simulink model "dds_cwg_32k_dual_test"  (R2016b) (continued)
== system dds_cwg_32k_dual_test/nb_pfb == (continued)
      mask Display:
      | port_label('input', 1, 'd');
      | port_label('output', 1, 'q');
      | disp(['Z^-', num2str(latency)]);
  pipeline61 : Subsystem  Commented=on  AncestorBlock=casper_library_delays/pipeline  mask(pipeline){latency=2}
      mask help:
      | An explicitly laid-out delay line to avoid mapping to SRL16s
      | for use in pipelining to help achieve timing closure.
      mask init:
      | pipeline_init(gcb,'latency',latency);
      settings: AttributesFormatString=Latency = %<latency>
      mask Display:
      | port_label('input', 1, 'd');
      | port_label('output', 1, 'q');
      | disp(['Z^-', num2str(latency)]);
  pipeline62 : Subsystem  AncestorBlock=casper_library_delays/pipeline  mask(pipeline){latency=2}
      mask help:
      | An explicitly laid-out delay line to avoid mapping to SRL16s
      | for use in pipelining to help achieve timing closure.
      mask init:
      | pipeline_init(gcb,'latency',latency);
      settings: AttributesFormatString=Latency = %<latency>
      mask Display:
      | port_label('input', 1, 'd');
      | port_label('output', 1, 'q');
      | disp(['Z^-', num2str(latency)]);
  pipeline63 : Subsystem  AncestorBlock=casper_library_delays/pipeline  mask(pipeline){latency=2}
      mask help:
      | An explicitly laid-out delay line to avoid mapping to SRL16s
      | for use in pipelining to help achieve timing closure.
      mask init:
      | pipeline_init(gcb,'latency',latency);
      settings: AttributesFormatString=Latency = %<latency>
      mask Display:
      | port_label('input', 1, 'd');
      | port_label('output', 1, 'q');
      | disp(['Z^-', num2str(latency)]);
  pipeline64 : Subsystem  AncestorBlock=casper_library_delays/pipeline  mask(pipeline){latency=2}
      mask help:
      | An explicitly laid-out delay line to avoid mapping to SRL16s
      | for use in pipelining to help achieve timing closure.
      mask init:
      | pipeline_init(gcb,'latency',latency);
      settings: AttributesFormatString=Latency = %<latency>
      mask Display:
      | port_label('input', 1, 'd');
      | port_label('output', 1, 'q');
      | disp(['Z^-', num2str(latency)]);
  pipeline65 : Subsystem  AncestorBlock=casper_library_delays/pipeline  mask(pipeline){latency=2}
      mask help:
      | An explicitly laid-out delay line to avoid mapping to SRL16s
      | for use in pipelining to help achieve timing closure.
      mask init:
      | pipeline_init(gcb,'latency',latency);
      settings: AttributesFormatString=Latency = %<latency>
      mask Display:
      | port_label('input', 1, 'd');
      | port_label('output', 1, 'q');
      | disp(['Z^-', num2str(latency)]);
  pipeline66 : Subsystem  AncestorBlock=casper_library_delays/pipeline  mask(pipeline){latency=2}
      mask help:
      | An explicitly laid-out delay line to avoid mapping to SRL16s
      | for use in pipelining to help achieve timing closure.
      mask init:
      | pipeline_init(gcb,'latency',latency);
      settings: AttributesFormatString=Latency = %<latency>
      mask Display:
      | port_label('input', 1, 'd');
      | port_label('output', 1, 'q');
      | disp(['Z^-', num2str(latency)]);
  pipeline67 : Subsystem  AncestorBlock=casper_library_delays/pipeline  mask(pipeline){latency=2}
      mask help:
      | An explicitly laid-out delay line to avoid mapping to SRL16s
      | for use in pipelining to help achieve timing closure.
      mask init:
      | pipeline_init(gcb,'latency',latency);
      settings: AttributesFormatString=Latency = %<latency>
      mask Display:
      | port_label('input', 1, 'd');
      | port_label('output', 1, 'q');
      | disp(['Z^-', num2str(latency)]);
  pipeline68 : Subsystem  AncestorBlock=casper_library_delays/pipeline  mask(pipeline){latency=2}
      mask help:
      | An explicitly laid-out delay line to avoid mapping to SRL16s
      | for use in pipelining to help achieve timing closure.
      mask init:
      | pipeline_init(gcb,'latency',latency);
      settings: AttributesFormatString=Latency = %<latency>
      mask Display:
      | port_label('input', 1, 'd');
      | port_label('output', 1, 'q');
      | disp(['Z^-', num2str(latency)]);
  pipeline69 : Subsystem  Commented=on  AncestorBlock=casper_library_delays/pipeline  mask(pipeline){latency=2}
      mask help:
      | An explicitly laid-out delay line to avoid mapping to SRL16s
      | for use in pipelining to help achieve timing closure.
      mask init:
      | pipeline_init(gcb,'latency',latency);
      settings: AttributesFormatString=Latency = %<latency>
      mask Display:
      | port_label('input', 1, 'd');
      | port_label('output', 1, 'q');
      | disp(['Z^-', num2str(latency)]);
  pipeline7 : Subsystem  AncestorBlock=casper_library_delays/pipeline  mask(pipeline){latency=2}
      mask help:
      | An explicitly laid-out delay line to avoid mapping to SRL16s
      | for use in pipelining to help achieve timing closure.
      mask init:
      | pipeline_init(gcb,'latency',latency);
      settings: AttributesFormatString=Latency = %<latency>
      mask Display:
      | port_label('input', 1, 'd');
      | port_label('output', 1, 'q');
      | disp(['Z^-', num2str(latency)]);
  pipeline70 : Subsystem  Commented=on  AncestorBlock=casper_library_delays/pipeline  mask(pipeline){latency=2}
      mask help:
      | An explicitly laid-out delay line to avoid mapping to SRL16s
      | for use in pipelining to help achieve timing closure.
      mask init:
      | pipeline_init(gcb,'latency',latency);
      settings: AttributesFormatString=Latency = %<latency>
      mask Display:
      | port_label('input', 1, 'd');
      | port_label('output', 1, 'q');
      | disp(['Z^-', num2str(latency)]);
  pipeline71 : Subsystem  AncestorBlock=casper_library_delays/pipeline  mask(pipeline){latency=2}
      mask help:
      | An explicitly laid-out delay line to avoid mapping to SRL16s
      | for use in pipelining to help achieve timing closure.
      mask init:
      | pipeline_init(gcb,'latency',latency);
      settings: AttributesFormatString=Latency = %<latency>
      mask Display:
      | port_label('input', 1, 'd');
      | port_label('output', 1, 'q');
      | disp(['Z^-', num2str(latency)]);
  pipeline72 : Subsystem  AncestorBlock=casper_library_delays/pipeline  mask(pipeline){latency=2}
      mask help:
      | An explicitly laid-out delay line to avoid mapping to SRL16s
      | for use in pipelining to help achieve timing closure.
      mask init:
      | pipeline_init(gcb,'latency',latency);
      settings: AttributesFormatString=Latency = %<latency>
      mask Display:
      | port_label('input', 1, 'd');
      | port_label('output', 1, 'q');
      | disp(['Z^-', num2str(latency)]);
  pipeline73 : Subsystem  AncestorBlock=casper_library_delays/pipeline  mask(pipeline){latency=2}
      mask help:
      | An explicitly laid-out delay line to avoid mapping to SRL16s
      | for use in pipelining to help achieve timing closure.
      mask init:
      | pipeline_init(gcb,'latency',latency);
      settings: AttributesFormatString=Latency = %<latency>
      mask Display:
      | port_label('input', 1, 'd');
      | port_label('output', 1, 'q');
      | disp(['Z^-', num2str(latency)]);
  pipeline74 : Subsystem  AncestorBlock=casper_library_delays/pipeline  mask(pipeline){latency=2}
      mask help:
      | An explicitly laid-out delay line to avoid mapping to SRL16s
      | for use in pipelining to help achieve timing closure.
      mask init:
      | pipeline_init(gcb,'latency',latency);
      settings: AttributesFormatString=Latency = %<latency>
      mask Display:
      | port_label('input', 1, 'd');
      | port_label('output', 1, 'q');
      | disp(['Z^-', num2str(latency)]);
  pipeline75 : Subsystem  AncestorBlock=casper_library_delays/pipeline  mask(pipeline){latency=2}
      mask help:
      | An explicitly laid-out delay line to avoid mapping to SRL16s
      | for use in pipelining to help achieve timing closure.
      mask init:
      | pipeline_init(gcb,'latency',latency);
      settings: AttributesFormatString=Latency = %<latency>
      mask Display:
      | port_label('input', 1, 'd');
      | port_label('output', 1, 'q');
      | disp(['Z^-', num2str(latency)]);
  pipeline76 : Subsystem  AncestorBlock=casper_library_delays/pipeline  mask(pipeline){latency=1}
      mask help:
      | An explicitly laid-out delay line to avoid mapping to SRL16s
      | for use in pipelining to help achieve timing closure.
      mask init:
      | pipeline_init(gcb,'latency',latency);
      settings: AttributesFormatString=Latency = %<latency>
      mask Display:
      | port_label('input', 1, 'd');
      | port_label('output', 1, 'q');
      | disp(['Z^-', num2str(latency)]);
  pipeline77 : Subsystem  AncestorBlock=casper_library_delays/pipeline  mask(pipeline){latency=2}
      mask help:
      | An explicitly laid-out delay line to avoid mapping to SRL16s
      | for use in pipelining to help achieve timing closure.
      mask init:
      | pipeline_init(gcb,'latency',latency);
      settings: AttributesFormatString=Latency = %<latency>
      mask Display:
      | port_label('input', 1, 'd');
      | port_label('output', 1, 'q');
      | disp(['Z^-', num2str(latency)]);
  pipeline78 : Subsystem  AncestorBlock=casper_library_delays/pipeline  mask(pipeline){latency=2}
      mask help:
      | An explicitly laid-out delay line to avoid mapping to SRL16s
      | for use in pipelining to help achieve timing closure.
      mask init:
      | pipeline_init(gcb,'latency',latency);
      settings: AttributesFormatString=Latency = %<latency>
      mask Display:
      | port_label('input', 1, 'd');
      | port_label('output', 1, 'q');
      | disp(['Z^-', num2str(latency)]);
  pipeline79 : Subsystem  Commented=on  AncestorBlock=casper_library_delays/pipeline  mask(pipeline){latency=2}
      mask help:
      | An explicitly laid-out delay line to avoid mapping to SRL16s
      | for use in pipelining to help achieve timing closure.
      mask init:
      | pipeline_init(gcb,'latency',latency);
      settings: AttributesFormatString=Latency = %<latency>
      mask Display:
      | port_label('input', 1, 'd');
      | port_label('output', 1, 'q');
      | disp(['Z^-', num2str(latency)]);
  pipeline8 : Subsystem  AncestorBlock=casper_library_delays/pipeline  mask(pipeline){latency=2}
      mask help:
      | An explicitly laid-out delay line to avoid mapping to SRL16s
      | for use in pipelining to help achieve timing closure.
      mask init:
      | pipeline_init(gcb,'latency',latency);
      settings: AttributesFormatString=Latency = %<latency>
      mask Display:
      | port_label('input', 1, 'd');
      | port_label('output', 1, 'q');
      | disp(['Z^-', num2str(latency)]);
  pipeline83 : Subsystem  AncestorBlock=casper_library_delays/pipeline  mask(pipeline){latency=2}
      mask help:
      | An explicitly laid-out delay line to avoid mapping to SRL16s
      | for use in pipelining to help achieve timing closure.
      mask init:
      | pipeline_init(gcb,'latency',latency);
      settings: AttributesFormatString=Latency = %<latency>
      mask Display:
      | port_label('input', 1, 'd');
      | port_label('output', 1, 'q');
      | disp(['Z^-', num2str(latency)]);
  pipeline9 : Subsystem  AncestorBlock=casper_library_delays/pipeline  mask(pipeline){latency=2}
      mask help:
      | An explicitly laid-out delay line to avoid mapping to SRL16s
      | for use in pipelining to help achieve timing closure.
      mask init:
      | pipeline_init(gcb,'latency',latency);
      settings: AttributesFormatString=Latency = %<latency>
      mask Display:
      | port_label('input', 1, 'd');
      | port_label('output', 1, 'q');
      | disp(['Z^-', num2str(latency)]);
  pipeline92 : Subsystem  AncestorBlock=casper_library_delays/pipeline  mask(pipeline){latency=2}
      mask help:
      | An explicitly laid-out delay line to avoid mapping to SRL16s
      | for use in pipelining to help achieve timing closure.
      mask init:
      | pipeline_init(gcb,'latency',latency);
      settings: AttributesFormatString=Latency = %<latency>
      mask Display:
      | port_label('input', 1, 'd');
      | port_label('output', 1, 'q');
      | disp(['Z^-', num2str(latency)]);
  pipeline93 : Subsystem  AncestorBlock=casper_library_delays/pipeline  mask(pipeline){latency=2}
      mask help:
      | An explicitly laid-out delay line to avoid mapping to SRL16s
      | for use in pipelining to help achieve timing closure.
      mask init:
      | pipeline_init(gcb,'latency',latency);
      settings: AttributesFormatString=Latency = %<latency>
      mask Display:
      | port_label('input', 1, 'd');
      | port_label('output', 1, 'q');
      | disp(['Z^-', num2str(latency)]);
  pipeline94 : Subsystem  AncestorBlock=casper_library_delays/pipeline  mask(pipeline){latency=2}
      mask help:
      | An explicitly laid-out delay line to avoid mapping to SRL16s
      | for use in pipelining to help achieve timing closure.
      mask init:
      | pipeline_init(gcb,'latency',latency);
      settings: AttributesFormatString=Latency = %<latency>
      mask Display:
      | port_label('input', 1, 'd');
      | port_label('output', 1, 'q');
      | disp(['Z^-', num2str(latency)]);
  pipeline95 : Subsystem  AncestorBlock=casper_library_delays/pipeline  mask(pipeline){latency=2}
      mask help:
      | An explicitly laid-out delay line to avoid mapping to SRL16s
      | for use in pipelining to help achieve timing closure.
      mask init:
      | pipeline_init(gcb,'latency',latency);
      settings: AttributesFormatString=Latency = %<latency>
      mask Display:
      | port_label('input', 1, 'd');
      | port_label('output', 1, 'q');
      | disp(['Z^-', num2str(latency)]);
  pipeline96 : Subsystem  AncestorBlock=casper_library_delays/pipeline  mask(pipeline){latency=2}
      mask help:
      | An explicitly laid-out delay line to avoid mapping to SRL16s
      | for use in pipelining to help achieve timing closure.
      mask init:
      | pipeline_init(gcb,'latency',latency);
      settings: AttributesFormatString=Latency = %<latency>
      mask Display:
      | port_label('input', 1, 'd');
      | port_label('output', 1, 'q');
      | disp(['Z^-', num2str(latency)]);
  pipeline97 : Subsystem  AncestorBlock=casper_library_delays/pipeline  mask(pipeline){latency=2}
      mask help:
      | An explicitly laid-out delay line to avoid mapping to SRL16s
      | for use in pipelining to help achieve timing closure.
      mask init:
      | pipeline_init(gcb,'latency',latency);
      settings: AttributesFormatString=Latency = %<latency>
      mask Display:
      | port_label('input', 1, 'd');
      | port_label('output', 1, 'q');
      | disp(['Z^-', num2str(latency)]);
  pipeline98 : Subsystem  AncestorBlock=casper_library_delays/pipeline  mask(pipeline){latency=2}
      mask help:
      | An explicitly laid-out delay line to avoid mapping to SRL16s
      | for use in pipelining to help achieve timing closure.
      mask init:
      | pipeline_init(gcb,'latency',latency);
      settings: AttributesFormatString=Latency = %<latency>
      mask Display:
      | port_label('input', 1, 'd');
      | port_label('output', 1, 'q');
      | disp(['Z^-', num2str(latency)]);
  pulse_ext : casper_library_misc/pulse_ext  pulse_len=10
  pulse_ext1 : casper_library_misc/pulse_ext  pulse_len=10
  register_change_detect : Subsystem  Commented=on
  ss_fft : Subsystem  Tag=casper:bitsnap  Commented=on  AncestorBlock=casper_library_scopes/bitfield_snapshot  mask(bitsnap){io_names=[re im ch dv sync of], io_widths=[18 18 15 1 1 1], io_bps=[0 0 0 0 0 0], io_types=[0 0 0 2 2 2], show_format=on, snap_storage=bram, snap_dram_dimm=2, snap_dram_clock=250, snap_nsamples=9, snap_data_width=64, snap_offset=on, snap_circap=off, snap_value=off, snap_ext_arm=off, snap_ext_circ=off, snap_provide_outputs=on, snap_use_dsp48=on, snap_delay=0, extra_names=[c d], extra_widths=[30 2], extra_bps=[0 0], extra_types=0}
      mask prompts: io_names "Names [msb...lsb]"; io_widths "Bitwidths [msb...lsb]"; io_bps "Binary pts [msb...lsb]"; io_types "Data types [msb...lsb], ufix=0, fix=1, bool=2"; show_format "Print format string?"; snap_storage "Storage medium"; snap_dram_dimm "DRAM dimm"; snap_dram_clock "DRAM clock rate"; snap_nsamples "Number of Samples (2^?)"; snap_data_width "Data width"; snap_offset "Start delay support"; snap_circap "Circular capture support"; snap_value "Extra value capture support"; snap_ext_arm "External arm support"; snap_ext_circ "External circular capture support"; snap_provide_outputs "Provide outputs"; snap_use_dsp48 "Use DSP48s to implement counters"; snap_delay "Pre-snapshot delay"; extra_names "Names [msb...lsb]"; extra_widths "Widths [msb...lsb]"; extra_bps "Binary pts [msb...lsb]"; extra_types "Data types [msb...lsb], ufix=0, fix=1, bool=2"
      mask help:
      | A wrapped snapshot block. Allows specification of inputs to a snapblock word so the toolflow can automatically set up the scope.
      | See documentation for snapshot for explanation of parameters.
      mask init:
      | bitsnap_init(gcb);
      settings: AttributesFormatString=|
      | snap(6):uf18.0,uf18.0,uf15.0,b,b,b = 54 bits\n64 wide, 512 deep
      | debugID: 42865123
      mask TypeOptions snap_storage: bram | dram
      mask Callback snap_storage: bitsnap_callback();
      mask TypeOptions snap_dram_dimm: 1 | 2 | 3 | 4
      mask TypeOptions snap_dram_clock: 200 | 250 | 266 | 300 | 333
      mask TypeOptions snap_data_width: 8 | 16 | 32 | 64 | 128
      mask Callback snap_value: bitsnap_callback();
      mask Callback snap_ext_arm: bitsnap_callback();
      mask Callback snap_ext_circ: bitsnap_callback();
      mask Callback snap_provide_outputs: bitsnap_callback();
  ss_fft3 : Subsystem  Tag=casper:bitsnap  Commented=on  AncestorBlock=casper_library_scopes/bitfield_snapshot  mask(bitsnap){io_names=[re im shift dv_in sync rst tlast dv_out ch0 re_ou sync_out dvu trdy err fifo], io_widths=[18 18 16 1 1 1 1 1 15 18 1 1 1 1 1], io_bps=[0 0 0 0 0 0 0 0 0 0 0 0 0 0 0], io_types=[0 0 0 2 2 2 2 2 0 0 2 2 2 2 2], show_format=on, snap_storage=bram, snap_dram_dimm=2, snap_dram_clock=250, snap_nsamples=10, snap_data_width=128, snap_offset=on, snap_circap=off, snap_value=off, snap_ext_arm=off, snap_ext_circ=off, snap_provide_outputs=on, snap_use_dsp48=on, snap_delay=0, extra_names=[c d], extra_widths=[30 2], extra_bps=[0 0], extra_types=0}
      mask prompts: io_names "Names [msb...lsb]"; io_widths "Bitwidths [msb...lsb]"; io_bps "Binary pts [msb...lsb]"; io_types "Data types [msb...lsb], ufix=0, fix=1, bool=2"; show_format "Print format string?"; snap_storage "Storage medium"; snap_dram_dimm "DRAM dimm"; snap_dram_clock "DRAM clock rate"; snap_nsamples "Number of Samples (2^?)"; snap_data_width "Data width"; snap_offset "Start delay support"; snap_circap "Circular capture support"; snap_value "Extra value capture support"; snap_ext_arm "External arm support"; snap_ext_circ "External circular capture support"; snap_provide_outputs "Provide outputs"; snap_use_dsp48 "Use DSP48s to implement counters"; snap_delay "Pre-snapshot delay"; extra_names "Names [msb...lsb]"; extra_widths "Widths [msb...lsb]"; extra_bps "Binary pts [msb...lsb]"; extra_types "Data types [msb...lsb], ufix=0, fix=1, bool=2"
      mask help:
      | A wrapped snapshot block. Allows specification of inputs to a snapblock word so the toolflow can automatically set up the scope.
      | See documentation for snapshot for explanation of parameters.
      mask init:
      | bitsnap_init(gcb);
      settings: AttributesFormatString=|
      | snap(15):uf18.0,uf18.0,uf16.0,b,b,b,b,b,uf15.0,uf18.0,b,b,b,b,b = 95 bits\n128 wide, 1024 deep
      | debugID: 120913192
      mask TypeOptions snap_storage: bram | dram
      mask Callback snap_storage: bitsnap_callback();
      mask TypeOptions snap_dram_dimm: 1 | 2 | 3 | 4
      mask TypeOptions snap_dram_clock: 200 | 250 | 266 | 300 | 333
      mask TypeOptions snap_data_width: 8 | 16 | 32 | 64 | 128
      mask Callback snap_value: bitsnap_callback();
      mask Callback snap_ext_arm: bitsnap_callback();
      mask Callback snap_ext_circ: bitsnap_callback();
      mask Callback snap_provide_outputs: bitsnap_callback();
  ss_fft4 : Subsystem  Tag=casper:bitsnap  Commented=on  AncestorBlock=casper_library_scopes/bitfield_snapshot  mask(bitsnap){io_names=[re_i re_o dv we rd sync empty full], io_widths=[18 18 1 1 1 1 1 1], io_bps=[0 0 0 0 0 0 0 0], io_types=[0 0 2 2 2 2 2 2], show_format=on, snap_storage=bram, snap_dram_dimm=2, snap_dram_clock=250, snap_nsamples=9, snap_data_width=64, snap_offset=on, snap_circap=off, snap_value=off, snap_ext_arm=off, snap_ext_circ=off, snap_provide_outputs=on, snap_use_dsp48=on, snap_delay=0, extra_names=[c d], extra_widths=[30 2], extra_bps=[0 0], extra_types=0}
      mask prompts: io_names "Names [msb...lsb]"; io_widths "Bitwidths [msb...lsb]"; io_bps "Binary pts [msb...lsb]"; io_types "Data types [msb...lsb], ufix=0, fix=1, bool=2"; show_format "Print format string?"; snap_storage "Storage medium"; snap_dram_dimm "DRAM dimm"; snap_dram_clock "DRAM clock rate"; snap_nsamples "Number of Samples (2^?)"; snap_data_width "Data width"; snap_offset "Start delay support"; snap_circap "Circular capture support"; snap_value "Extra value capture support"; snap_ext_arm "External arm support"; snap_ext_circ "External circular capture support"; snap_provide_outputs "Provide outputs"; snap_use_dsp48 "Use DSP48s to implement counters"; snap_delay "Pre-snapshot delay"; extra_names "Names [msb...lsb]"; extra_widths "Widths [msb...lsb]"; extra_bps "Binary pts [msb...lsb]"; extra_types "Data types [msb...lsb], ufix=0, fix=1, bool=2"
      mask help:
      | A wrapped snapshot block. Allows specification of inputs to a snapblock word so the toolflow can automatically set up the scope.
      | See documentation for snapshot for explanation of parameters.
      mask init:
      | bitsnap_init(gcb);
      settings: AttributesFormatString=|
      | snap(8):uf18.0,uf18.0,b,b,b,b,b,b = 42 bits\n64 wide, 512 deep
      | debugID: 120942983
      mask TypeOptions snap_storage: bram | dram
      mask Callback snap_storage: bitsnap_callback();
      mask TypeOptions snap_dram_dimm: 1 | 2 | 3 | 4
      mask TypeOptions snap_dram_clock: 200 | 250 | 266 | 300 | 333
      mask TypeOptions snap_data_width: 8 | 16 | 32 | 64 | 128
      mask Callback snap_value: bitsnap_callback();
      mask Callback snap_ext_arm: bitsnap_callback();
      mask Callback snap_ext_circ: bitsnap_callback();
      mask Callback snap_provide_outputs: bitsnap_callback();
  ss_fft_debug : Subsystem  Tag=casper:bitsnap  Commented=on  AncestorBlock=casper_library_scopes/bitfield_snapshot  mask(bitsnap){io_names=[fft0_re fft0_im fft1_re fft1_im trdy0 ch0 dv0 sync0 of0 trdy1 ch1 dv1 of1], io_widths=[18 18 18 18 1 15 1 1 1 1 15 1 1], io_bps=[17 17 17 17 0 0 0 0 0 0 0 0 0], io_types=[1 1 1 1 2 0 2 2 2 2 0 2 2], show_format=on, snap_storage=bram, snap_dram_dimm=2, snap_dram_clock=250, snap_nsamples=15, snap_data_width=128, snap_offset=on, snap_circap=off, snap_value=off, snap_ext_arm=off, snap_ext_circ=off, snap_provide_outputs=on, snap_use_dsp48=on, snap_delay=0, extra_names=[c d], extra_widths=[30 2], extra_bps=[0 0], extra_types=0}
      mask prompts: io_names "Names [msb...lsb]"; io_widths "Bitwidths [msb...lsb]"; io_bps "Binary pts [msb...lsb]"; io_types "Data types [msb...lsb], ufix=0, fix=1, bool=2"; show_format "Print format string?"; snap_storage "Storage medium"; snap_dram_dimm "DRAM dimm"; snap_dram_clock "DRAM clock rate"; snap_nsamples "Number of Samples (2^?)"; snap_data_width "Data width"; snap_offset "Start delay support"; snap_circap "Circular capture support"; snap_value "Extra value capture support"; snap_ext_arm "External arm support"; snap_ext_circ "External circular capture support"; snap_provide_outputs "Provide outputs"; snap_use_dsp48 "Use DSP48s to implement counters"; snap_delay "Pre-snapshot delay"; extra_names "Names [msb...lsb]"; extra_widths "Widths [msb...lsb]"; extra_bps "Binary pts [msb...lsb]"; extra_types "Data types [msb...lsb], ufix=0, fix=1, bool=2"
      mask help:
      | A wrapped snapshot block. Allows specification of inputs to a snapblock word so the toolflow can automatically set up the scope.
      | See documentation for snapshot for explanation of parameters.
      mask init:
      | bitsnap_init(gcb);
      settings: AttributesFormatString=|
      | snap(13):f18.17,f18.17,f18.17,f18.17,b,uf15.0,b,b,b,b,uf15.0,b,b = 109 bits\n128 wide, 32768 deep
      | debugID: 34277647
      mask TypeOptions snap_storage: bram | dram
      mask Callback snap_storage: bitsnap_callback();
      mask TypeOptions snap_dram_dimm: 1 | 2 | 3 | 4
      mask TypeOptions snap_dram_clock: 200 | 250 | 266 | 300 | 333
      mask TypeOptions snap_data_width: 8 | 16 | 32 | 64 | 128
      mask Callback snap_value: bitsnap_callback();
      mask Callback snap_ext_arm: bitsnap_callback();
      mask Callback snap_ext_circ: bitsnap_callback();
      mask Callback snap_provide_outputs: bitsnap_callback();
  ss_fft_debug1 : Subsystem  Tag=casper:bitsnap  Commented=on  AncestorBlock=casper_library_scopes/bitfield_snapshot  mask(bitsnap){io_names=[fft0_re fft0_im fft1_re fft1_im trdy0 ch0 dv0 sync0 of0 trdy1 ch1 dv1 of1], io_widths=[18 18 18 18 1 15 1 1 1 1 15 1 1], io_bps=[17 17 17 17 0 0 0 0 0 0 0 0 0], io_types=[1 1 1 1 2 0 2 2 2 2 0 2 2], show_format=on, snap_storage=bram, snap_dram_dimm=2, snap_dram_clock=250, snap_nsamples=15, snap_data_width=128, snap_offset=on, snap_circap=off, snap_value=off, snap_ext_arm=off, snap_ext_circ=off, snap_provide_outputs=on, snap_use_dsp48=on, snap_delay=0, extra_names=[c d], extra_widths=[30 2], extra_bps=[0 0], extra_types=0}
      mask prompts: io_names "Names [msb...lsb]"; io_widths "Bitwidths [msb...lsb]"; io_bps "Binary pts [msb...lsb]"; io_types "Data types [msb...lsb], ufix=0, fix=1, bool=2"; show_format "Print format string?"; snap_storage "Storage medium"; snap_dram_dimm "DRAM dimm"; snap_dram_clock "DRAM clock rate"; snap_nsamples "Number of Samples (2^?)"; snap_data_width "Data width"; snap_offset "Start delay support"; snap_circap "Circular capture support"; snap_value "Extra value capture support"; snap_ext_arm "External arm support"; snap_ext_circ "External circular capture support"; snap_provide_outputs "Provide outputs"; snap_use_dsp48 "Use DSP48s to implement counters"; snap_delay "Pre-snapshot delay"; extra_names "Names [msb...lsb]"; extra_widths "Widths [msb...lsb]"; extra_bps "Binary pts [msb...lsb]"; extra_types "Data types [msb...lsb], ufix=0, fix=1, bool=2"
      mask help:
      | A wrapped snapshot block. Allows specification of inputs to a snapblock word so the toolflow can automatically set up the scope.
      | See documentation for snapshot for explanation of parameters.
      mask init:
      | bitsnap_init(gcb);
      settings: AttributesFormatString=|
      | snap(13):f18.17,f18.17,f18.17,f18.17,b,uf15.0,b,b,b,b,uf15.0,b,b = 109 bits\n128 wide, 32768 deep
      | debugID: 123077310
      mask TypeOptions snap_storage: bram | dram
      mask Callback snap_storage: bitsnap_callback();
      mask TypeOptions snap_dram_dimm: 1 | 2 | 3 | 4
      mask TypeOptions snap_dram_clock: 200 | 250 | 266 | 300 | 333
      mask TypeOptions snap_data_width: 8 | 16 | 32 | 64 | 128
      mask Callback snap_value: bitsnap_callback();
      mask Callback snap_ext_arm: bitsnap_callback();
      mask Callback snap_ext_circ: bitsnap_callback();
      mask Callback snap_provide_outputs: bitsnap_callback();
  ss_fir_out : Subsystem  Tag=casper:bitsnap  Commented=on  AncestorBlock=casper_library_scopes/bitfield_snapshot  mask(bitsnap){io_names=[fir_re fir_im dv sync], io_widths=[18 18 1 1], io_bps=[0 17 0 0], io_types=[0 1 2 2], show_format=on, snap_storage=bram, snap_dram_dimm=2, snap_dram_clock=250, snap_nsamples=9, snap_data_width=64, snap_offset=on, snap_circap=off, snap_value=off, snap_ext_arm=off, snap_ext_circ=off, snap_provide_outputs=on, snap_use_dsp48=on, snap_delay=0, extra_names=[c d], extra_widths=[30 2], extra_bps=[0 0], extra_types=0}
      mask prompts: io_names "Names [msb...lsb]"; io_widths "Bitwidths [msb...lsb]"; io_bps "Binary pts [msb...lsb]"; io_types "Data types [msb...lsb], ufix=0, fix=1, bool=2"; show_format "Print format string?"; snap_storage "Storage medium"; snap_dram_dimm "DRAM dimm"; snap_dram_clock "DRAM clock rate"; snap_nsamples "Number of Samples (2^?)"; snap_data_width "Data width"; snap_offset "Start delay support"; snap_circap "Circular capture support"; snap_value "Extra value capture support"; snap_ext_arm "External arm support"; snap_ext_circ "External circular capture support"; snap_provide_outputs "Provide outputs"; snap_use_dsp48 "Use DSP48s to implement counters"; snap_delay "Pre-snapshot delay"; extra_names "Names [msb...lsb]"; extra_widths "Widths [msb...lsb]"; extra_bps "Binary pts [msb...lsb]"; extra_types "Data types [msb...lsb], ufix=0, fix=1, bool=2"
      mask help:
      | A wrapped snapshot block. Allows specification of inputs to a snapblock word so the toolflow can automatically set up the scope.
      | See documentation for snapshot for explanation of parameters.
      mask init:
      | bitsnap_init(gcb);
      settings: AttributesFormatString=|
      | snap(4):uf18.0,f18.17,b,b = 38 bits\n64 wide, 512 deep
      | debugID: 22068793
      mask TypeOptions snap_storage: bram | dram
      mask Callback snap_storage: bitsnap_callback();
      mask TypeOptions snap_dram_dimm: 1 | 2 | 3 | 4
      mask TypeOptions snap_dram_clock: 200 | 250 | 266 | 300 | 333
      mask TypeOptions snap_data_width: 8 | 16 | 32 | 64 | 128
      mask Callback snap_value: bitsnap_callback();
      mask Callback snap_ext_arm: bitsnap_callback();
      mask Callback snap_ext_circ: bitsnap_callback();
      mask Callback snap_provide_outputs: bitsnap_callback();
  sync_out : Outport
  dv_out : Outport  Port=2
  tag scope: fft_ch0=global, fft_ch1=global, fft_conf_tready_p0=global, fft_dv0=global, fft_dv1=global, fft_p0_fifo_full=global, fft_pol0_err=global, fft_pol0_of=global, fft_pol1_err=global, fft_pol1_of=global, fft_sync0=global, fft_tready_p0=global, fir_data_tlast=global, fir_dvalid_pol0=global, fir_pol0=global, fir_sync=global
  wires:
  pipeline29/1(q) -> ss_fft_debug/5(in_trdy0)
  pipeline30/1(q) -> ss_fft_debug/6(in_ch0)
  pipeline31/1(q) -> ss_fft_debug/7(in_dv0)
  pipeline33/1(q) -> ss_fft_debug/8(in_sync0)
  pipeline26/1(q) -> ss_fft_debug/9(in_of0)
  pipeline34/1(q) -> ss_fft_debug/10(in_trdy1)
  pipeline32/1(q) -> ss_fft_debug/11(in_ch1)
  pipeline15/1(q) -> ss_fft_debug/15(trig)
  pipeline35/1(q) -> ss_fft_debug/12(in_dv1)
  pipeline51/1(q) -> ss_fft_debug/13(in_of1)
  pipeline52/1(q) -> ss_fft_debug/14(we)
  From68/1 -> pipeline34/1(d)
  From72/1 -> pipeline29/1(d)
  From73/1 -> pipeline30/1(d)
  From74/1 -> pipeline52/1(d), pipeline31/1(d)
  From75/1 -> pipeline15/1(d), pipeline33/1(d)
  From76/1 -> pipeline26/1(d)
  From77/1 -> pipeline51/1(d)
  From78/1 -> pipeline32/1(d)
  From80/1 -> pipeline35/1(d)
  pipeline1/1(q) -> ss_fft_debug/4(in_fft1_im)
  pipeline2/1(q) -> ss_fft_debug/3(in_fft1_re)
  pipeline27/1(q) -> ss_fft_debug/2(in_fft0_im)
  pipeline28/1(q) -> ss_fft_debug/1(in_fft0_re)
  From2/1 -> pipeline27/1(d)
  From1/1 -> pipeline28/1(d)
  From5/1 -> pipeline2/1(d)
  From6/1 -> pipeline1/1(d)
  pipeline45/1(q) -> ss_fft_debug1/5(in_trdy0)
  pipeline49/1(q) -> ss_fft_debug1/6(in_ch0)
  pipeline54/1(q) -> ss_fft_debug1/7(in_dv0)
  pipeline56/1(q) -> ss_fft_debug1/8(in_sync0)
  pipeline41/1(q) -> ss_fft_debug1/9(in_of0)
  pipeline57/1(q) -> ss_fft_debug1/10(in_trdy1)
  pipeline55/1(q) -> ss_fft_debug1/11(in_ch1)
  pipeline38/1(q) -> ss_fft_debug1/15(trig)
  pipeline58/1(q) -> ss_fft_debug1/12(in_dv1)
  pipeline59/1(q) -> ss_fft_debug1/13(in_of1)
  pipeline60/1(q) -> ss_fft_debug1/14(we)
  From17/1 -> pipeline57/1(d)
  From18/1 -> pipeline45/1(d)
  From19/1 -> pipeline49/1(d)
  From26/1 -> pipeline54/1(d), pipeline60/1(d)
  From20/1 -> pipeline56/1(d), pipeline38/1(d)
  From22/1 -> pipeline41/1(d)
  From23/1 -> pipeline59/1(d)
  From24/1 -> pipeline55/1(d)
  From25/1 -> pipeline58/1(d)
  pipeline37/1(q) -> ss_fft_debug1/4(in_fft1_im)
  pipeline40/1(q) -> ss_fft_debug1/3(in_fft1_re)
  pipeline42/1(q) -> ss_fft_debug1/2(in_fft0_im)
  pipeline43/1(q) -> ss_fft_debug1/1(in_fft0_re)
  From11/1 -> pipeline42/1(d)
  From8/1 -> pipeline43/1(d)
  From14/1 -> pipeline40/1(d)
  From15/1 -> pipeline37/1(d)
  [fft_sync0] -> sync_out/1
  [fft_dv0] -> dv_out/1
  pfb_fir_generic0/2(pol0_out0) -> pipeline18/1(d)
  pfb_fir_generic1/2(pol0_out0) -> pipeline20/1(d)
  pfb_fir_generic0/1(sync_out) -> pipeline17/1(d)
  pfb_fir_generic1/1(sync_out) -> Terminator5/1
  pol1/1 -> pfb_fir_generic1/2(pol0_in0)
  Gateway Out6/1 -> To Workspace6/1
  Gateway Out1/1 -> To Workspace1/1
  Gateway Out9/1 -> To Workspace9/1
  Gateway Out36/1 -> To Workspace36/1
  Gateway Out39/1 -> To Workspace39/1
  pfb_fir_generic0/3(dvalid) -> pipeline19/1(d)
  pfb_fir_generic1/3(dvalid) -> pipeline21/1(d)
  sync/1 -> pfb_fir_generic0/1(sync), pfb_fir_generic1/1(sync), Gateway Out9/1
  Counter3/1 -> Relational4/1, Gateway Out53/1
  Constant9/1 -> Relational4/2
  dvalid/1 -> pfb_fir_generic0/3(en), pfb_fir_generic1/3(en), Gateway Out36/1
  Gateway Out70/1 -> To Workspace69/1
  Gateway Out71/1 -> To Workspace70/1
  [fft_re_pol0] -> Gateway Out70/1
  [fft_im_pol0] -> Gateway Out71/1
  fft_shift/1 -> [fft_shift]
  Constant2/1 -> Mux3/2
  [fft_dv0] -> Mux3/1
  Mux3/1 -> [fft_re_pol0]
  Constant1/1 -> Mux4/2
  Mux4/1 -> [fft_im_pol0]
  [fft_re_pol0_int] -> Mux3/3
  [fft_im_pol0_int] -> Mux4/3
  [fft_dv0] -> Mux4/1
  pipeline19/1(q) -> [fir_dvalid_pol0], Gateway Out1/1, Counter3/2
  pipeline20/1(q) -> Goto16/1
  pipeline21/1(q) -> Goto18/1
  pipeline18/1(q) -> [fir_pol0]
  [cnt_rst] -> pipeline230/1(d)
  Counter12/1 -> fir_sync_cnt/1(out_reg)
  pipeline229/1(q) -> Counter12/2
  pipeline230/1(q) -> Counter12/1
  "Fast Fourier Transform 9.0 "/1 -> pipeline23/1(d)
  pipeline117/1(q) -> [fft_tready_p0]
  pipeline110/1(q) -> [fft_ch0]
  Gateway Out66/1 -> To Workspace65/1
  Gateway Out67/1 -> To Workspace66/1
  Gateway Out72/1 -> To Workspace71/1
  [fft_dv0] -> Gateway Out67/1
  [fft_ch0] -> Gateway Out66/1
  [fft_tready_p0] -> Gateway Out72/1
  "Fast Fourier Transform 9.0 "/5 -> pipeline122/1(d)
  "Fast Fourier Transform 9.0 "/6 -> pipeline123/1(d)
  "Fast Fourier Transform 9.0 "/8 -> pipeline110/1(d), Relational2/1
  "Fast Fourier Transform 9.0 "/15 -> Terminator7/1
  "Fast Fourier Transform 9.0 "/16 -> Terminator8/1
  "Fast Fourier Transform 9.0 "/17 -> Terminator9/1
  pipeline123/1(q) -> [fft_re_pol0_int]
  pipeline122/1(q) -> [fft_im_pol0_int]
  Inverter1/1 -> "Fast Fourier Transform 9.0 "/10, Logical9/2, pipeline64/1(d)
  Gateway Out37/1 -> To Workspace37/1
  [fft_sync0] -> Gateway Out37/1
  From79/1 -> Delay84/1
  Inverter2/1 -> Fast Fourier Transform 9.0 2/10, Logical2/2
  Logical7/1 -> Inverter2/1
  Constant7/1 -> Mux9/2
  [fft_dv1] -> Mux9/1
  Mux9/1 -> [fft_re_pol1]
  Constant6/1 -> Mux10/2
  Mux10/1 -> [fft_im_pol1]
  [fft_re_pol1_int] -> Mux9/3
  [fft_im_pol1_int] -> Mux10/3
  [fft_dv1] -> Mux10/1
  Constant12/1 -> "Fast Fourier Transform 9.0 "/8
  Constant10/1 -> "Fast Fourier Transform 9.0 "/3
  Constant3/1 -> "Fast Fourier Transform 9.0 "/2
  Constant13/1 -> Fast Fourier Transform 9.0 2/3
  Delay81/1 -> Fast Fourier Transform 9.0 2/4
  Delay82/1 -> Fast Fourier Transform 9.0 2/5
  Delay83/1 -> Logical2/1
  Logical2/1 -> Fast Fourier Transform 9.0 2/6
  From118/1 -> Delay83/1
  Delay84/1 -> Fast Fourier Transform 9.0 2/7
  Constant8/1 -> Fast Fourier Transform 9.0 2/9
  pipeline124/1(q) -> [fft_dv0]
  edge_detect3/1(out) -> [fft_sync0]
  edge_detect1/1(out) -> Register5/2
  Register5/1 -> edge_detect3/1(in)
  From47/1 -> bus_expand11/1(bus_in)
  [fir_pol0] -> bus_expand13/1(bus_in)
  Gateway Out4/1 -> To Workspace4/1
  bus_expand13/1(msb_out2) -> Gateway Out4/1
  Gateway Out8/1 -> To Workspace8/1
  bus_expand13/2(lsb_out1) -> Gateway Out8/1
  bus_expand11/2(lsb_out1) -> Delay81/1
  bus_expand11/1(msb_out2) -> Delay82/1
  bus_expand14/1(msb_out2) -> Gateway Out39/1
  Gateway Out35/1 -> To Workspace35/1
  bus_expand14/2(lsb_out1) -> Gateway Out35/1
  Gateway Out48/1 -> To Workspace53/1
  Gateway Out53/1 -> To Workspace54/1
  edge_detect9/1(out) -> Gateway Out48/1, [fir_data_tlast]
  Relational4/1 -> edge_detect9/1(in)
  pipeline17/1(q) -> edge_detect10/1(in), Counter3/1
  From12/1 -> Delay98/1, Logical7/1
  Delay98/1 -> Logical7/2
  [fir_sync] -> pipeline229/1(d)
  edge_detect10/1(out) -> [fir_sync], Gateway Out6/1
  Constant/1 -> Relational2/2
  Relational2/1 -> Logical/1
  Logical/1 -> edge_detect1/1(in)
  Delay109/1 -> Logical/2
  Delay9/1 -> Inverter4/1, Register5/1, Register5/3
  Inverter4/1 -> Logical/3
  Fast Fourier Transform 9.0 2/1 -> Terminator1/1
  Fast Fourier Transform 9.0 2/10 -> Terminator12/1
  Fast Fourier Transform 9.0 2/17 -> Terminator4/1
  Fast Fourier Transform 9.0 2/16 -> Terminator3/1
  Fast Fourier Transform 9.0 2/15 -> Terminator2/1
  Fast Fourier Transform 9.0 2/13 -> Terminator11/1
  Fast Fourier Transform 9.0 2/11 -> Terminator13/1
  Fast Fourier Transform 9.0 2/12 -> Terminator21/1
  Fast Fourier Transform 9.0 2/2 -> Terminator14/1
  Fast Fourier Transform 9.0 2/9 -> pipeline50/1(d)
  Fast Fourier Transform 9.0 2/8 -> pipeline12/1(d)
  Fast Fourier Transform 9.0 2/6 -> pipeline48/1(d)
  Fast Fourier Transform 9.0 2/5 -> pipeline44/1(d)
  Constant5/1 -> Fast Fourier Transform 9.0 2/2
  Fast Fourier Transform 9.0 2/3 -> Terminator15/1
  Fast Fourier Transform 9.0 2/4 -> Terminator16/1
  Constant4/1 -> "Fast Fourier Transform 9.0 "/9
  Constant11/1 -> Fast Fourier Transform 9.0 2/8
  "Fast Fourier Transform 9.0 "/3 -> Terminator17/1
  "Fast Fourier Transform 9.0 "/4 -> Terminator18/1
  "Fast Fourier Transform 9.0 "/13 -> Terminator20/1
  "Fast Fourier Transform 9.0 "/12 -> Terminator22/1
  "Fast Fourier Transform 9.0 "/11 -> Terminator23/1
  "Fast Fourier Transform 9.0 "/10 -> Terminator24/1
  pipeline16/1(q) -> [fft_pol0_of]
  From361/1 -> register_change_detect/1(data)
  register_change_detect/1(state_change) -> edge_detect7/1(in)
  edge_detect7/1(out) -> Goto38/1
  "Fast Fourier Transform 9.0 "/7 -> Terminator19/1
  "Fast Fourier Transform 9.0 "/14 -> pipeline16/1(d)
  Fast Fourier Transform 9.0 2/14 -> pipeline5/1(d)
  Fast Fourier Transform 9.0 2/7 -> Terminator10/1
  Counter2/1 -> fft_shift_change/1(out_reg)
  pipeline3/1(q) -> Counter2/1
  From45/1 -> pipeline3/1(d)
  pipeline22/1(q) -> Counter2/2
  From46/1 -> pipeline22/1(d)
  pipeline23/1(q) -> [fft_conf_tready_p0]
  From109/1 -> Slice/1
  From13/1 -> Slice1/1
  Slice/1 -> Concat4/2
  Constant14/1 -> Concat4/1
  Slice1/1 -> Concat6/2
  Constant15/1 -> Concat6/1
  Concat6/1 -> Fast Fourier Transform 9.0 2/1
  From43/1 -> Mux2/2
  Concat4/1 -> Mux2/3
  Constant27/1 -> fft_shift_sel/1(sim_1)
  fft_shift_sel/1(in_sel) -> Mux2/1
  From50/1 -> pipeline79/1(d), pipeline69/1(d)
  pipeline69/1(q) -> ss_fir_out/3(in_dv)
  pipeline70/1(q) -> ss_fir_out/4(in_sync)
  pipeline79/1(q) -> ss_fir_out/5(we)
  pipeline25/1(q) -> ss_fir_out/6(trig)
  From16/1 -> pipeline25/1(d), pipeline70/1(d)
  bus_expand12/2(lsb_out1) -> pipeline61/1(d)
  bus_expand12/1(msb_out2) -> pipeline10/1(d)
  From44/1 -> bus_expand12/1(bus_in)
  pipeline61/1(q) -> ss_fir_out/2(in_fir_im)
  pipeline10/1(q) -> Reinterpret10/1
  From58/1 -> pipeline36/1(d)
  From67/1 -> pipeline24/1(d)
  From42/1 -> pipeline39/1(d)
  From55/1 -> pipeline4/1(d), pipeline46/1(d)
  From59/1 -> pipeline47/1(d)
  pipeline24/1(q) -> Reinterpret8/1
  pipeline36/1(q) -> Reinterpret9/1
  pipeline39/1(q) -> ss_fft/3(in_ch)
  pipeline46/1(q) -> ss_fft/4(in_dv)
  pipeline47/1(q) -> ss_fft/6(in_of)
  pipeline4/1(q) -> ss_fft/7(we)
  pipeline11/1(q) -> ss_fft/8(trig)
  From66/1 -> pipeline13/1(d), pipeline11/1(d)
  pipeline13/1(q) -> ss_fft/5(in_sync)
  [fft_im_pol0_int] -> [fft_im_pol1_int]
  [fft_re_pol0_int] -> [fft_re_pol1_int]
  [fft_ch0] -> [fft_ch1]
  [fft_dv0] -> [fft_dv1]
  [fft_pol0_of] -> [fft_pol1_of]
  Reinterpret8/1 -> ss_fft/1(in_re)
  Reinterpret9/1 -> ss_fft/2(in_im)
  Reinterpret10/1 -> ss_fir_out/1(in_fir_re)
  [fft_shift] -> "Fast Fourier Transform 9.0 "/1
  Mux2/1 -> Terminator6/1
  [fft_shift] -> pipeline53/1(d)
  pipeline8/1(q) -> Reinterpret11/1
  pipeline9/1(q) -> Reinterpret12/1
  pipeline62/1(q) -> ss_fft3/16(we)
  pipeline6/1(q) -> ss_fft3/17(trig)
  pipeline7/1(q) -> ss_fft3/5(in_sync)
  Reinterpret11/1 -> ss_fft3/1(in_re)
  Reinterpret12/1 -> ss_fft3/2(in_im)
  pipeline53/1(q) -> ss_fft3/3(in_shift)
  [fir_sync] -> pipeline6/1(d), pipeline7/1(d)
  pipeline64/1(q) -> ss_fft3/6(in_rst)
  pipeline65/1(q) -> ss_fft3/8(in_dv_out)
  pipeline66/1(q) -> ss_fft3/7(in_tlast)
  [fft_dv0] -> pipeline65/1(d)
  pipeline68/1(q) -> ss_fft3/9(in_ch0)
  [fft_ch0] -> pipeline68/1(d)
  [fft_re_pol0_int] -> pipeline67/1(d)
  pipeline67/1(q) -> Reinterpret14/1
  pipeline71/1(q) -> ss_fft3/11(in_sync_out)
  [fft_sync0] -> pipeline71/1(d)
  "Fast Fourier Transform 9.0 "/9 -> Logical9/1, pipeline72/1(d)
  pipeline72/1(q) -> ss_fft3/12(in_dvu)
  pipeline63/1(q) -> ss_fft3/4(in_dv_in)
  Logical9/1 -> pipeline124/1(d), Delay109/1
  [fft_tready_p0] -> edge_detect4/1(in)
  Counter6/1 -> fft_tready_cnt0/1(out_reg)
  edge_detect4/1(out) -> pipeline78/1(d)
  pipeline73/1(q) -> Counter6/1
  [cnt_rst] -> pipeline73/1(d)
  pipeline74/1(q) -> ss_fft3/13(in_trdy)
  "Fast Fourier Transform 9.0 "/2 -> pipeline74/1(d), pipeline117/1(d), Inverter5/1
  bus_expand6/1(msb_out2) -> "Fast Fourier Transform 9.0 "/5, pipeline8/1(d)
  bus_expand6/2(lsb_out1) -> "Fast Fourier Transform 9.0 "/4, pipeline9/1(d)
  FIFO/1 -> bus_expand1/1(bus_in), bus_expand10/1(bus_in)
  [fir_data_tlast] -> "Fast Fourier Transform 9.0 "/7, pipeline66/1(d)
  Logical12/1 -> [fft_pol0_err]
  pipeline75/1(q) -> ss_fft3/14(in_err)
  [fft_pol0_err] -> pipeline75/1(d)
  Inverter5/1 -> Logical12/1
  pipeline78/1(q) -> Counter6/2
  pulse_ext/1 -> Inverter1/1
  Reinterpret14/1 -> ss_fft3/10(in_re_ou)
  Constant16/1 -> [fft_pol1_err]
  FIFO/3 -> pipeline96/1(d), [fft_p0_fifo_full]
  pulse_ext1/1 -> Inverter7/1, pipeline76/1(d), pipeline77/1(d), FIFO/4
  Logical1/1 -> Delay1/1, pipeline95/1(d), FIFO/2
  Inverter7/1 -> Logical1/2
  Delay2/1 -> pipeline94/1(d), pipeline93/1(d), Logical1/1
  Delay6/1 -> FIFO/1, bus_expand7/1(bus_in)
  pipeline14/1(q) -> ss_fft3/15(in_fifo)
  [fft_p0_fifo_full] -> pipeline14/1(d)
  pipeline83/1(q) -> Reinterpret13/1
  pipeline92/1(q) -> Reinterpret15/1
  pipeline94/1(q) -> ss_fft4/9(we)
  pipeline76/1(q) -> ss_fft4/10(trig)
  pipeline77/1(q) -> ss_fft4/6(in_sync)
  pipeline96/1(q) -> ss_fft4/8(in_full)
  Reinterpret13/1 -> ss_fft4/1(in_re_i)
  Reinterpret15/1 -> ss_fft4/2(in_re_o)
  pipeline93/1(q) -> ss_fft4/3(in_dv)
  pipeline95/1(q) -> ss_fft4/4(in_we)
  pipeline97/1(q) -> ss_fft4/5(in_rd)
  pipeline98/1(q) -> ss_fft4/7(in_empty)
  bus_expand7/1(msb_out2) -> pipeline83/1(d)
  bus_expand10/1(msb_out2) -> pipeline92/1(d)
  [fft_tready_p0] -> Delay11/1, pipeline97/1(d), FIFO/3
  Constant17/1 -> bus_expand6/1(bus_in)
  Gateway Out2/1 -> To Workspace2/1
  Gateway Out3/1 -> To Workspace3/1
  Gateway Out5/1 -> To Workspace5/1
  bus_expand1/2(lsb_out1) -> Gateway Out5/1
  Gateway Out7/1 -> To Workspace7/1
  bus_expand1/1(msb_out2) -> Gateway Out7/1
  FIFO/2 -> Logical11/3, Gateway Out10/1, Inverter8/1, Gateway Out3/1, pipeline98/1(d)
  pol0/1 -> pfb_fir_generic0/2(pol0_in0), bus_expand14/1(bus_in)
  [fir_pol0] -> Delay6/1
  [fir_dvalid_pol0] -> Delay2/1
  [fir_sync] -> pulse_ext1/1
  [fir_sync] -> Delay9/1, pulse_ext/1
  Gateway Out10/1 -> To Workspace10/1
  Logical13/1 -> "Fast Fourier Transform 9.0 "/6, Logical12/2, pipeline62/1(d), pipeline63/1(d)
  Logical11/1 -> Logical13/1
  Logical14/1 -> Logical13/2
  Delay11/1 -> Logical11/2, Logical14/2
  [fft_p0_fifo_full] -> Gateway Out2/1
  Inverter8/1 -> Logical14/1
  Delay1/1 -> Logical11/1
